simulink model "SOC_uncommented"  (R2023b) (continued)
== system SOC_uncommented/SOC == (continued)
  Multi-Temperature SOC EKF69 : Subsystem  TreatAsAtomicUnit=on  SystemSampleTime=1
  Multi-Temperature SOC EKF7 : Subsystem  TreatAsAtomicUnit=on  SystemSampleTime=1
  Multi-Temperature SOC EKF70 : Subsystem  TreatAsAtomicUnit=on  SystemSampleTime=1
  Multi-Temperature SOC EKF71 : Subsystem  TreatAsAtomicUnit=on  SystemSampleTime=1
  Multi-Temperature SOC EKF72 : Subsystem  TreatAsAtomicUnit=on  SystemSampleTime=1
  Multi-Temperature SOC EKF73 : Subsystem  TreatAsAtomicUnit=on  SystemSampleTime=1
  Multi-Temperature SOC EKF74 : Subsystem  TreatAsAtomicUnit=on  SystemSampleTime=1
  Multi-Temperature SOC EKF75 : Subsystem  TreatAsAtomicUnit=on  SystemSampleTime=1
  Multi-Temperature SOC EKF76 : Subsystem  TreatAsAtomicUnit=on  SystemSampleTime=1
  Multi-Temperature SOC EKF77 : Subsystem  TreatAsAtomicUnit=on  SystemSampleTime=1
  Multi-Temperature SOC EKF78 : Subsystem  TreatAsAtomicUnit=on  SystemSampleTime=1
  Multi-Temperature SOC EKF79 : Subsystem  TreatAsAtomicUnit=on  SystemSampleTime=1
  Multi-Temperature SOC EKF8 : Subsystem  TreatAsAtomicUnit=on  SystemSampleTime=1
  Multi-Temperature SOC EKF80 : Subsystem  TreatAsAtomicUnit=on  SystemSampleTime=1
  Multi-Temperature SOC EKF81 : Subsystem  TreatAsAtomicUnit=on  SystemSampleTime=1
  Multi-Temperature SOC EKF82 : Subsystem  TreatAsAtomicUnit=on  SystemSampleTime=1
  Multi-Temperature SOC EKF83 : Subsystem  TreatAsAtomicUnit=on  SystemSampleTime=1
  Multi-Temperature SOC EKF84 : Subsystem  TreatAsAtomicUnit=on  SystemSampleTime=1
  Multi-Temperature SOC EKF85 : Subsystem  TreatAsAtomicUnit=on  SystemSampleTime=1
  Multi-Temperature SOC EKF86 : Subsystem  TreatAsAtomicUnit=on  SystemSampleTime=1
  Multi-Temperature SOC EKF87 : Subsystem  TreatAsAtomicUnit=on  SystemSampleTime=1
  Multi-Temperature SOC EKF88 : Subsystem  TreatAsAtomicUnit=on  SystemSampleTime=1
  Multi-Temperature SOC EKF89 : Subsystem  TreatAsAtomicUnit=on  SystemSampleTime=1
  Multi-Temperature SOC EKF9 : Subsystem  TreatAsAtomicUnit=on  SystemSampleTime=1
  Multi-Temperature SOC EKF90 : Subsystem  TreatAsAtomicUnit=on  SystemSampleTime=1
  Multi-Temperature SOC EKF91 : Subsystem  TreatAsAtomicUnit=on  SystemSampleTime=1
  Multi-Temperature SOC EKF92 : Subsystem  TreatAsAtomicUnit=on  SystemSampleTime=1
  Multi-Temperature SOC EKF93 : Subsystem  TreatAsAtomicUnit=on  SystemSampleTime=1
  Multi-Temperature SOC EKF94 : Subsystem  TreatAsAtomicUnit=on  SystemSampleTime=1
  Multi-Temperature SOC EKF95 : Subsystem  TreatAsAtomicUnit=on  SystemSampleTime=1
  Multi-Temperature SOC EKF96 : Subsystem  TreatAsAtomicUnit=on  SystemSampleTime=1
  Multi-Temperature SOC EKF97 : Subsystem  TreatAsAtomicUnit=on  SystemSampleTime=1
  Multi-Temperature SOC EKF98 : Subsystem  TreatAsAtomicUnit=on  SystemSampleTime=1
  Multi-Temperature SOC EKF99 : Subsystem  TreatAsAtomicUnit=on  SystemSampleTime=1
  tag scope: HVBatSOC=global, HVBatSOCMax=global, HVBatSOCMin=global, HVBat_SOCcells=global
  wires:
  Bus Selector2/63 -> Multi-Temperature SOC EKF63/3(Temperature)  "<Cell Temperature 63>"
  Bus Selector2/81 -> Multi-Temperature SOC EKF81/3(Temperature)  "<Cell Temperature 81>"
  Bus Selector2/101 -> Multi-Temperature SOC EKF101/3(Temperature)  "<Cell Temperature 101>"
  [HVBatCell_Voltage042] -> Multi-Temperature SOC EKF42/2(Voltage)
  [HVBatCell_Voltage095] -> Multi-Temperature SOC EKF95/2(Voltage)
  Bus Selector2/70 -> Multi-Temperature SOC EKF70/3(Temperature)  "<Cell Temperature 70>"
  Multi-Temperature SOC EKF76/1(HVBat_SOCcell076) -> Bus Creator2/76  "HVBat_SOCcell076"
  [HVBatCell_Voltage055] -> Multi-Temperature SOC EKF55/2(Voltage)
  Bus Selector2/82 -> Multi-Temperature SOC EKF82/3(Temperature)  "<Cell Temperature 82>"
  Bus Selector2/83 -> Multi-Temperature SOC EKF83/3(Temperature)  "<Cell Temperature 83>"
  Bus Selector2/85 -> Multi-Temperature SOC EKF85/3(Temperature)  "<Cell Temperature 85>"
  Bus Selector2/10 -> Multi-Temperature SOC EKF10/3(Temperature)  "<Cell Temperature 10>"
  [HVBatCell_Voltage077] -> Multi-Temperature SOC EKF77/2(Voltage)
  [HVBatCell_Voltage088] -> Multi-Temperature SOC EKF88/2(Voltage)
  Multi-Temperature SOC EKF28/1(HVBat_SOCcell028) -> Bus Creator2/28  "HVBat_SOCcell028"
  [HVBatCell_Voltage020] -> Multi-Temperature SOC EKF20/2(Voltage)
  Bus Selector2/100 -> Multi-Temperature SOC EKF100/3(Temperature)  "<Cell Temperature 100>"
  Multi-Temperature SOC EKF12/1(HVBat_SOCcell012) -> Bus Creator2/12  "HVBat_SOCcell012"
  [HVBatCell_Voltage089] -> Multi-Temperature SOC EKF89/2(Voltage)
  Bus Selector2/18 -> Multi-Temperature SOC EKF18/3(Temperature)  "<Cell Temperature 18>"
  [HVBatCell_Voltage029] -> Multi-Temperature SOC EKF29/2(Voltage)
  [HVBatCell_Voltage015] -> Multi-Temperature SOC EKF15/2(Voltage)
  [HVBatCell_Voltage075] -> Multi-Temperature SOC EKF75/2(Voltage)
  Bus Selector2/20 -> Multi-Temperature SOC EKF20/3(Temperature)  "<Cell Temperature 20>"
  [HVBatCell_Voltage102] -> Multi-Temperature SOC EKF102/2(Voltage)
  [HVBatCell_Voltage091] -> Multi-Temperature SOC EKF91/2(Voltage)
  Multi-Temperature SOC EKF41/1(HVBat_SOCcell041) -> Bus Creator2/41  "HVBat_SOCcell041"
  Bus Selector2/71 -> Multi-Temperature SOC EKF71/3(Temperature)  "<Cell Temperature 71>"
  [HVBatCell_Voltage092] -> Multi-Temperature SOC EKF92/2(Voltage)
  Multi-Temperature SOC EKF50/1(HVBat_SOCcell050) -> Bus Creator2/50  "HVBat_SOCcell050"
  Multi-Temperature SOC EKF15/1(HVBat_SOCcell015) -> Bus Creator2/15  "HVBat_SOCcell015"
  [HVBatCell_Voltage048] -> Multi-Temperature SOC EKF48/2(Voltage)
  [HVBatCell_Voltage041] -> Multi-Temperature SOC EKF41/2(Voltage)
  Bus Selector2/49 -> Multi-Temperature SOC EKF49/3(Temperature)  "<Cell Temperature 49>"
  Bus Selector2/50 -> Multi-Temperature SOC EKF50/3(Temperature)  "<Cell Temperature 50>"
  [HVBatCell_Voltage093] -> Multi-Temperature SOC EKF93/2(Voltage)
  Bus Selector2/37 -> Multi-Temperature SOC EKF37/3(Temperature)  "<Cell Temperature 37>"
  Bus Selector2/2 -> Multi-Temperature SOC EKF2/3(Temperature)  "<Cell Temperature 2>"
  Gain1/1 -> Multi-Temperature SOC EKF2/1(Current), Multi-Temperature SOC EKF34/1(Current), Multi-Temperature SOC EKF35/1(Current), Multi-Temperature SOC EKF39/1(Current), Multi-Temperature SOC EKF105/1(Current), Multi-Temperature SOC EKF104/1(Current), Multi-Temperature SOC EKF103/1(Current), Multi-Temperature SOC EKF102/1(Current), Multi-Temperature SOC EKF101/1(Current), Multi-Temperature SOC EKF100/1(Current), Multi-Temperature SOC EKF99/1(Current), Multi-Temperature SOC EKF98/1(Current), Multi-Temperature SOC EKF97/1(Current), Multi-Temperature SOC EKF96/1(Current), Multi-Temperature SOC EKF95/1(Current), Multi-Temperature SOC EKF94/1(Current), Multi-Temperature SOC EKF93/1(Current), Multi-Temperature SOC EKF92/1(Current), Multi-Temperature SOC EKF91/1(Current), Multi-Temperature SOC EKF90/1(Current), Multi-Temperature SOC EKF89/1(Current), Multi-Temperature SOC EKF88/1(Current), Multi-Temperature SOC EKF87/1(Current), Multi-Temperature SOC EKF86/1(Current), Multi-Temperature SOC EKF85/1(Current), Multi-Temperature SOC EKF84/1(Current), Multi-Temperature SOC EKF83/1(Current), Multi-Temperature SOC EKF82/1(Current), Multi-Temperature SOC EKF81/1(Current), Multi-Temperature SOC EKF80/1(Current), Multi-Temperature SOC EKF79/1(Current), Multi-Temperature SOC EKF78/1(Current), Multi-Temperature SOC EKF77/1(Current), Multi-Temperature SOC EKF76/1(Current), Multi-Temperature SOC EKF75/1(Current), Multi-Temperature SOC EKF74/1(Current), Multi-Temperature SOC EKF73/1(Current), Multi-Temperature SOC EKF72/1(Current), Multi-Temperature SOC EKF71/1(Current), Multi-Temperature SOC EKF70/1(Current), Multi-Temperature SOC EKF69/1(Current), Multi-Temperature SOC EKF68/1(Current), Multi-Temperature SOC EKF67/1(Current), Multi-Temperature SOC EKF66/1(Current), Multi-Temperature SOC EKF65/1(Current), Multi-Temperature SOC EKF64/1(Current), Multi-Temperature SOC EKF63/1(Current), Multi-Temperature SOC EKF62/1(Current), Multi-Temperature SOC EKF61/1(Current), Multi-Temperature SOC EKF60/1(Current), Multi-Temperature SOC EKF59/1(Current), Multi-Temperature SOC EKF58/1(Current), Multi-Temperature SOC EKF57/1(Current), Multi-Temperature SOC EKF56/1(Current), Multi-Temperature SOC EKF55/1(Current), Multi-Temperature SOC EKF54/1(Current), Multi-Temperature SOC EKF53/1(Current), Multi-Temperature SOC EKF52/1(Current), Multi-Temperature SOC EKF51/1(Current), Multi-Temperature SOC EKF50/1(Current), Multi-Temperature SOC EKF49/1(Current), Multi-Temperature SOC EKF48/1(Current), Multi-Temperature SOC EKF47/1(Current), Multi-Temperature SOC EKF46/1(Current), Multi-Temperature SOC EKF45/1(Current), Multi-Temperature SOC EKF44/1(Current), Multi-Temperature SOC EKF43/1(Current), Multi-Temperature SOC EKF42/1(Current), Multi-Temperature SOC EKF41/1(Current), Multi-Temperature SOC EKF40/1(Current), Multi-Temperature SOC EKF38/1(Current), Multi-Temperature SOC EKF37/1(Current), Multi-Temperature SOC EKF36/1(Current), Multi-Temperature SOC EKF33/1(Current), Multi-Temperature SOC EKF32/1(Current), Multi-Temperature SOC EKF31/1(Current), Multi-Temperature SOC EKF30/1(Current), Multi-Temperature SOC EKF29/1(Current), Multi-Temperature SOC EKF28/1(Current), Multi-Temperature SOC EKF27/1(Current), Multi-Temperature SOC EKF26/1(Current), Multi-Temperature SOC EKF25/1(Current), Multi-Temperature SOC EKF24/1(Current), Multi-Temperature SOC EKF23/1(Current), Multi-Temperature SOC EKF22/1(Current), Multi-Temperature SOC EKF21/1(Current), Multi-Temperature SOC EKF20/1(Current), Multi-Temperature SOC EKF19/1(Current), Multi-Temperature SOC EKF18/1(Current), Multi-Temperature SOC EKF17/1(Current), Multi-Temperature SOC EKF16/1(Current), Multi-Temperature SOC EKF15/1(Current), Multi-Temperature SOC EKF14/1(Current), Multi-Temperature SOC EKF13/1(Current), Multi-Temperature SOC EKF12/1(Current), Multi-Temperature SOC EKF11/1(Current), Multi-Temperature SOC EKF10/1(Current), Multi-Temperature SOC EKF9/1(Current), Multi-Temperature SOC EKF8/1(Current), Multi-Temperature SOC EKF7/1(Current), Multi-Temperature SOC EKF6/1(Current), Multi-Temperature SOC EKF5/1(Current), Multi-Temperature SOC EKF4/1(Current), Multi-Temperature SOC EKF3/1(Current), Multi-Temperature SOC EKF1/1(Current)  "CellCurrent"
  Multi-Temperature SOC EKF102/1(HVBat_SOCcell102) -> Bus Creator2/102  "HVBat_SOCcell102"
  Multi-Temperature SOC EKF54/1(HVBat_SOCcell054) -> Bus Creator2/54  "HVBat_SOCcell054"
  Bus Selector2/75 -> Multi-Temperature SOC EKF75/3(Temperature)  "<Cell Temperature 75>"
  [HVBatCell_Voltage007] -> Multi-Temperature SOC EKF7/2(Voltage)
  Multi-Temperature SOC EKF11/1(HVBat_SOCcell011) -> Bus Creator2/11  "HVBat_SOCcell011"
  [HVBatCell_Voltage087] -> Multi-Temperature SOC EKF87/2(Voltage)
  Multi-Temperature SOC EKF4/1(HVBat_SOCcell004) -> Bus Creator2/4  "HVBat_SOCcell004"
  [HVBatCell_Voltage037] -> Multi-Temperature SOC EKF37/2(Voltage)
  Bus Selector2/57 -> Multi-Temperature SOC EKF57/3(Temperature)  "<Cell Temperature 57>"
  [HVBatCell_Voltage083] -> Multi-Temperature SOC EKF83/2(Voltage)
  Bus Selector2/43 -> Multi-Temperature SOC EKF43/3(Temperature)  "<Cell Temperature 43>"
  [HVBatCell_Voltage035] -> Multi-Temperature SOC EKF35/2(Voltage)
  [HVBatCell_Voltage053] -> Multi-Temperature SOC EKF53/2(Voltage)
  Multi-Temperature SOC EKF35/1(HVBat_SOCcell035) -> Bus Creator2/35  "HVBat_SOCcell035"
  Multi-Temperature SOC EKF65/1(HVBat_SOCcell065) -> Bus Creator2/65  "HVBat_SOCcell065"
  Multi-Temperature SOC EKF73/1(HVBat_SOCcell073) -> Bus Creator2/73  "HVBat_SOCcell073"
  [HVBatCell_Voltage001] -> Multi-Temperature SOC EKF1/2(Voltage)
  [HVBatCell_Voltage084] -> Multi-Temperature SOC EKF84/2(Voltage)
  [HVBatCell_Voltage098] -> Multi-Temperature SOC EKF98/2(Voltage)
  Bus Selector2/62 -> Multi-Temperature SOC EKF62/3(Temperature)  "<Cell Temperature 62>"
  [HVBatCell_Voltage034] -> Multi-Temperature SOC EKF34/2(Voltage)
  Bus Selector2/5 -> Multi-Temperature SOC EKF5/3(Temperature)  "<Cell Temperature 5>"
  Multi-Temperature SOC EKF67/1(HVBat_SOCcell067) -> Bus Creator2/67  "HVBat_SOCcell067"
  [HVBatCell_Temperatures] -> Bus Selector2/1
  [HVBatCell_Voltage104] -> Multi-Temperature SOC EKF104/2(Voltage)
  Bus Selector2/3 -> Multi-Temperature SOC EKF3/3(Temperature)  "<Cell Temperature 3>"
  Bus Selector2/29 -> Multi-Temperature SOC EKF29/3(Temperature)  "<Cell Temperature 29>"
  Multi-Temperature SOC EKF78/1(HVBat_SOCcell078) -> Bus Creator2/78  "HVBat_SOCcell078"
  Multi-Temperature SOC EKF104/1(HVBat_SOCcell104) -> Bus Creator2/104  "HVBat_SOCcell104"
  Multi-Temperature SOC EKF46/1(HVBat_SOCcell046) -> Bus Creator2/46  "HVBat_SOCcell046"
  Bus Selector2/95 -> Multi-Temperature SOC EKF95/3(Temperature)  "<Cell Temperature 95>"
  [HVBatCell_Voltage023] -> Multi-Temperature SOC EKF23/2(Voltage)
  [HVBatCell_Voltage019] -> Multi-Temperature SOC EKF19/2(Voltage)
  Bus Selector2/51 -> Multi-Temperature SOC EKF51/3(Temperature)  "<Cell Temperature 51>"
  [HVBatCell_Voltage078] -> Multi-Temperature SOC EKF78/2(Voltage)
  Bus Selector2/65 -> Multi-Temperature SOC EKF65/3(Temperature)  "<Cell Temperature 65>"
  Multi-Temperature SOC EKF6/1(HVBat_SOCcell006) -> Bus Creator2/6  "HVBat_SOCcell006"
  Multi-Temperature SOC EKF103/1(HVBat_SOCcell103) -> Bus Creator2/103  "HVBat_SOCcell103"
  Bus Selector2/64 -> Multi-Temperature SOC EKF64/3(Temperature)  "<Cell Temperature 64>"
  [HVBatCell_Voltage052] -> Multi-Temperature SOC EKF52/2(Voltage)
  Multi-Temperature SOC EKF38/1(HVBat_SOCcell038) -> Bus Creator2/38  "HVBat_SOCcell038"
  Multi-Temperature SOC EKF30/1(HVBat_SOCcell030) -> Bus Creator2/30  "HVBat_SOCcell030"
  [HVBatCell_Voltage054] -> Multi-Temperature SOC EKF54/2(Voltage)
  Bus Selector2/45 -> Multi-Temperature SOC EKF45/3(Temperature)  "<Cell Temperature 45>"
  [HVBatCell_Voltage081] -> Multi-Temperature SOC EKF81/2(Voltage)
  Bus Selector2/26 -> Multi-Temperature SOC EKF26/3(Temperature)  "<Cell Temperature 26>"
  Bus Selector2/4 -> Multi-Temperature SOC EKF4/3(Temperature)  "<Cell Temperature 4>"
  [HVBatCell_Voltage049] -> Multi-Temperature SOC EKF49/2(Voltage)
  Multi-Temperature SOC EKF95/1(HVBat_SOCcell095) -> Bus Creator2/95  "HVBat_SOCcell095"
  Multi-Temperature SOC EKF100/1(HVBat_SOCcell100) -> Bus Creator2/100  "HVBat_SOCcell100"
  [HVBatCell_Voltage067] -> Multi-Temperature SOC EKF67/2(Voltage)
  [HVBatCell_Voltage069] -> Multi-Temperature SOC EKF69/2(Voltage)
  Bus Selector2/12 -> Multi-Temperature SOC EKF12/3(Temperature)  "<Cell Temperature 12>"
  Bus Selector2/93 -> Multi-Temperature SOC EKF93/3(Temperature)  "<Cell Temperature 93>"
  Multi-Temperature SOC EKF7/1(HVBat_SOCcell007) -> Bus Creator2/7  "HVBat_SOCcell007"
  Bus Selector2/91 -> Multi-Temperature SOC EKF91/3(Temperature)  "<Cell Temperature 91>"
  [HVBatCell_Voltage099] -> Multi-Temperature SOC EKF99/2(Voltage)
  [HVBatCell_Voltage032] -> Multi-Temperature SOC EKF32/2(Voltage)
  [HVBatCell_Voltage051] -> Multi-Temperature SOC EKF51/2(Voltage)
  Multi-Temperature SOC EKF16/1(HVBat_SOCcell016) -> Bus Creator2/16  "HVBat_SOCcell016"
  [HVBatCell_Voltage080] -> Multi-Temperature SOC EKF80/2(Voltage)
  [HVBatCell_Voltage021] -> Multi-Temperature SOC EKF21/2(Voltage)
  Multi-Temperature SOC EKF2/1(HVBat_SOCcell002) -> Bus Creator2/2  "HVBat_SOCcell002"
  Multi-Temperature SOC EKF27/1(HVBat_SOCcell027) -> Bus Creator2/27  "HVBat_SOCcell027"
  Bus Selector2/72 -> Multi-Temperature SOC EKF72/3(Temperature)  "<Cell Temperature 72>"
  Bus Selector2/80 -> Multi-Temperature SOC EKF80/3(Temperature)  "<Cell Temperature 80>"
  [HVBatCell_Voltage031] -> Multi-Temperature SOC EKF31/2(Voltage)
  [HVBatCell_Voltage045] -> Multi-Temperature SOC EKF45/2(Voltage)
  MATLAB Function1/2(HVBat_Energy) -> Data Store Write3/1
  Bus Selector2/56 -> Multi-Temperature SOC EKF56/3(Temperature)  "<Cell Temperature 56>"
  [HVBatCell_Voltage047] -> Multi-Temperature SOC EKF47/2(Voltage)
  Bus Selector2/97 -> Multi-Temperature SOC EKF97/3(Temperature)  "<Cell Temperature 97>"
  Bus Selector2/44 -> Multi-Temperature SOC EKF44/3(Temperature)  "<Cell Temperature 44>"
  Multi-Temperature SOC EKF47/1(HVBat_SOCcell047) -> Bus Creator2/47  "HVBat_SOCcell047"
  Bus Selector2/74 -> Multi-Temperature SOC EKF74/3(Temperature)  "<Cell Temperature 74>"
  Bus Selector2/90 -> Multi-Temperature SOC EKF90/3(Temperature)  "<Cell Temperature 90>"
  [HVBatCell_Voltage003] -> Multi-Temperature SOC EKF3/2(Voltage)
  Multi-Temperature SOC EKF86/1(HVBat_SOCcell086) -> Bus Creator2/86  "HVBat_SOCcell086"
  Bus Selector2/98 -> Multi-Temperature SOC EKF98/3(Temperature)  "<Cell Temperature 98>"
  Multi-Temperature SOC EKF20/1(HVBat_SOCcell020) -> Bus Creator2/20  "HVBat_SOCcell020"
  Multi-Temperature SOC EKF13/1(HVBat_SOCcell013) -> Bus Creator2/13  "HVBat_SOCcell013"
  [HVBatCell_Voltage100] -> Multi-Temperature SOC EKF100/2(Voltage)
  [HVBatCell_Voltage074] -> Multi-Temperature SOC EKF74/2(Voltage)
  Multi-Temperature SOC EKF19/1(HVBat_SOCcell019) -> Bus Creator2/19  "HVBat_SOCcell019"
  [HVBatCell_Voltage009] -> Multi-Temperature SOC EKF9/2(Voltage)
  Multi-Temperature SOC EKF39/1(HVBat_SOCcell039) -> Bus Creator2/39  "HVBat_SOCcell039"
  [HVBatCell_Voltage070] -> Multi-Temperature SOC EKF70/2(Voltage)
  Max1/1 -> [HVBatSOCMin], MATLAB Function/2(HVBatSOCMin)  "HVBatSOCMin"
  [HVBatCell_Voltage094] -> Multi-Temperature SOC EKF94/2(Voltage)
  Multi-Temperature SOC EKF5/1(HVBat_SOCcell005) -> Bus Creator2/5  "HVBat_SOCcell005"
  [HVBatCell_Voltage050] -> Multi-Temperature SOC EKF50/2(Voltage)
  Bus Selector2/52 -> Multi-Temperature SOC EKF52/3(Temperature)  "<Cell Temperature 52>"
  [HVBatCell_Voltage059] -> Multi-Temperature SOC EKF59/2(Voltage)
  [HVBatCell_Voltage010] -> Multi-Temperature SOC EKF10/2(Voltage)
  Multi-Temperature SOC EKF25/1(HVBat_SOCcell025) -> Bus Creator2/25  "HVBat_SOCcell025"
  [HVBatCell_Voltage013] -> Multi-Temperature SOC EKF13/2(Voltage)
  Bus Selector2/99 -> Multi-Temperature SOC EKF99/3(Temperature)  "<Cell Temperature 99>"
  Multi-Temperature SOC EKF105/1(HVBat_SOCcell105) -> Bus Creator2/105  "HVBat_SOCcell105"
  [HVBatCell_Voltage011] -> Multi-Temperature SOC EKF11/2(Voltage)
  Multi-Temperature SOC EKF18/1(HVBat_SOCcell018) -> Bus Creator2/18  "HVBat_SOCcell018"
  Multi-Temperature SOC EKF43/1(HVBat_SOCcell043) -> Bus Creator2/43  "HVBat_SOCcell043"
  Multi-Temperature SOC EKF68/1(HVBat_SOCcell068) -> Bus Creator2/68  "HVBat_SOCcell068"
  Bus Selector2/67 -> Multi-Temperature SOC EKF67/3(Temperature)  "<Cell Temperature 67>"
  Multi-Temperature SOC EKF98/1(HVBat_SOCcell098) -> Bus Creator2/98  "HVBat_SOCcell098"
  [HVBatCell_Voltage097] -> Multi-Temperature SOC EKF97/2(Voltage)
  Multi-Temperature SOC EKF94/1(HVBat_SOCcell094) -> Bus Creator2/94  "HVBat_SOCcell094"
  [HVBatCell_Voltage026] -> Multi-Temperature SOC EKF26/2(Voltage)
  Multi-Temperature SOC EKF42/1(HVBat_SOCcell042) -> Bus Creator2/42  "HVBat_SOCcell042"
  Multi-Temperature SOC EKF90/1(HVBat_SOCcell090) -> Bus Creator2/90  "HVBat_SOCcell090"
  Bus Selector2/68 -> Multi-Temperature SOC EKF68/3(Temperature)  "<Cell Temperature 68>"
  Multi-Temperature SOC EKF93/1(HVBat_SOCcell093) -> Bus Creator2/93  "HVBat_SOCcell093"
  Multi-Temperature SOC EKF91/1(HVBat_SOCcell091) -> Bus Creator2/91  "HVBat_SOCcell091"
  Multi-Temperature SOC EKF87/1(HVBat_SOCcell087) -> Bus Creator2/87  "HVBat_SOCcell087"
  [HVBatCell_Voltage016] -> Multi-Temperature SOC EKF16/2(Voltage)
  [HVBatCell_Voltage096] -> Multi-Temperature SOC EKF96/2(Voltage)
  Multi-Temperature SOC EKF34/1(HVBat_SOCcell034) -> Bus Creator2/34  "HVBat_SOCcell034"
  Multi-Temperature SOC EKF62/1(HVBat_SOCcell062) -> Bus Creator2/62  "HVBat_SOCcell062"
  Multi-Temperature SOC EKF45/1(HVBat_SOCcell045) -> Bus Creator2/45  "HVBat_SOCcell045"
  Multi-Temperature SOC EKF60/1(HVBat_SOCcell060) -> Bus Creator2/60  "HVBat_SOCcell060"
  [HVBatCell_Voltage027] -> Multi-Temperature SOC EKF27/2(Voltage)
  Multi-Temperature SOC EKF26/1(HVBat_SOCcell026) -> Bus Creator2/26  "HVBat_SOCcell026"
  Multi-Temperature SOC EKF8/1(HVBat_SOCcell008) -> Bus Creator2/8  "HVBat_SOCcell008"
  [HVBatCell_Voltage082] -> Multi-Temperature SOC EKF82/2(Voltage)
  [HVBatCell_Voltage073] -> Multi-Temperature SOC EKF73/2(Voltage)
  Multi-Temperature SOC EKF17/1(HVBat_SOCcell017) -> Bus Creator2/17  "HVBat_SOCcell017"
  Multi-Temperature SOC EKF88/1(HVBat_SOCcell088) -> Bus Creator2/88  "HVBat_SOCcell088"
  Bus Selector2/104 -> Multi-Temperature SOC EKF104/3(Temperature)  "<Cell Temperature 87>"
  Multi-Temperature SOC EKF69/1(HVBat_SOCcell069) -> Bus Creator2/69  "HVBat_SOCcell069"
  Bus Selector2/69 -> Multi-Temperature SOC EKF69/3(Temperature)  "<Cell Temperature 69>"
  Bus Selector2/34 -> Multi-Temperature SOC EKF34/3(Temperature)  "<Cell Temperature 34>"
  [HVBatCell_Voltage105] -> Multi-Temperature SOC EKF105/2(Voltage)
  Bus Selector2/61 -> Multi-Temperature SOC EKF61/3(Temperature)  "<Cell Temperature 61>"
  Multi-Temperature SOC EKF23/1(HVBat_SOCcell023) -> Bus Creator2/23  "HVBat_SOCcell023"
  Bus Selector2/84 -> Multi-Temperature SOC EKF84/3(Temperature)  "<Cell Temperature 84>"
  [HVBatCell_Voltage064] -> Multi-Temperature SOC EKF64/2(Voltage)
  Bus Selector2/60 -> Multi-Temperature SOC EKF60/3(Temperature)  "<Cell Temperature 60>"
  Bus Selector2/59 -> Multi-Temperature SOC EKF59/3(Temperature)  "<Cell Temperature 59>"
  Multi-Temperature SOC EKF79/1(HVBat_SOCcell079) -> Bus Creator2/79  "HVBat_SOCcell079"
  Multi-Temperature SOC EKF75/1(HVBat_SOCcell075) -> Bus Creator2/75  "HVBat_SOCcell075"
  Bus Selector2/103 -> Multi-Temperature SOC EKF103/3(Temperature)  "<Cell Temperature 103>"
  Bus Selector2/24 -> Multi-Temperature SOC EKF24/3(Temperature)  "<Cell Temperature 24>"
  Multi-Temperature SOC EKF14/1(HVBat_SOCcell014) -> Bus Creator2/14  "HVBat_SOCcell014"
  [HVBatCell_Voltage079] -> Multi-Temperature SOC EKF79/2(Voltage)
  Max4/1 -> [HVBatSOCMax], MATLAB Function/1(HVBatSOCMax)  "HVBatSOCMax"
  [HVBatCell_Voltage057] -> Multi-Temperature SOC EKF57/2(Voltage)
  Bus Selector2/27 -> Multi-Temperature SOC EKF27/3(Temperature)  "<Cell Temperature 27>"
  Bus Selector2/14 -> Multi-Temperature SOC EKF14/3(Temperature)  "<Cell Temperature 14>"
  Multi-Temperature SOC EKF81/1(HVBat_SOCcell081) -> Bus Creator2/81  "HVBat_SOCcell081"
  Multi-Temperature SOC EKF29/1(HVBat_SOCcell029) -> Bus Creator2/29  "HVBat_SOCcell029"
  Bus Selector2/39 -> Multi-Temperature SOC EKF39/3(Temperature)  "<Cell Temperature 39>"
  Multi-Temperature SOC EKF33/1(HVBat_SOCcell033) -> Bus Creator2/33  "HVBat_SOCcell033"
  Multi-Temperature SOC EKF92/1(HVBat_SOCcell092) -> Bus Creator2/92  "HVBat_SOCcell092"
  Bus Selector2/30 -> Multi-Temperature SOC EKF30/3(Temperature)  "<Cell Temperature 30>"
  [HVBatCell_Voltage038] -> Multi-Temperature SOC EKF38/2(Voltage)
  Bus Selector2/89 -> Multi-Temperature SOC EKF89/3(Temperature)  "<Cell Temperature 89>"
  Bus Selector2/36 -> Multi-Temperature SOC EKF36/3(Temperature)  "<Cell Temperature 36>"
  Multi-Temperature SOC EKF22/1(HVBat_SOCcell022) -> Bus Creator2/22  "HVBat_SOCcell022"
  Bus Selector2/55 -> Multi-Temperature SOC EKF55/3(Temperature)  "<Cell Temperature 55>"
  Multi-Temperature SOC EKF84/1(HVBat_SOCcell084) -> Bus Creator2/84  "HVBat_SOCcell084"
  Multi-Temperature SOC EKF9/1(HVBat_SOCcell009) -> Bus Creator2/9  "HVBat_SOCcell009"
  Multi-Temperature SOC EKF85/1(HVBat_SOCcell085) -> Bus Creator2/85  "HVBat_SOCcell085"
  Bus Creator2/1 -> Max1/1, Max4/1, [HVBat_SOCcells]
  Bus Selector2/73 -> Multi-Temperature SOC EKF73/3(Temperature)  "<Cell Temperature 73>"
  Multi-Temperature SOC EKF3/1(HVBat_SOCcell003) -> Bus Creator2/3  "HVBat_SOCcell003"
  Multi-Temperature SOC EKF77/1(HVBat_SOCcell077) -> Bus Creator2/77  "HVBat_SOCcell077"
  Multi-Temperature SOC EKF48/1(HVBat_SOCcell048) -> Bus Creator2/48  "HVBat_SOCcell048"
  Multi-Temperature SOC EKF37/1(HVBat_SOCcell037) -> Bus Creator2/37  "HVBat_SOCcell037"
  [HVBatCell_Voltage043] -> Multi-Temperature SOC EKF43/2(Voltage)
  Bus Selector2/46 -> Multi-Temperature SOC EKF46/3(Temperature)  "<Cell Temperature 46>"
  [HVBatPack_Current_Prim] -> Gain1/1  "HVBatPack_Current_Prim"
  [HVBatCell_Voltage086] -> Multi-Temperature SOC EKF86/2(Voltage)
  Multi-Temperature SOC EKF56/1(HVBat_SOCcell056) -> Bus Creator2/56  "HVBat_SOCcell056"
  Multi-Temperature SOC EKF71/1(HVBat_SOCcell071) -> Bus Creator2/71  "HVBat_SOCcell071"
  [HVBatCell_Voltage036] -> Multi-Temperature SOC EKF36/2(Voltage)
  [HVBatCell_Voltage025] -> Multi-Temperature SOC EKF25/2(Voltage)
  [HVBatCell_Voltage044] -> Multi-Temperature SOC EKF44/2(Voltage)
  Multi-Temperature SOC EKF59/1(HVBat_SOCcell059) -> Bus Creator2/59  "HVBat_SOCcell059"
  Multi-Temperature SOC EKF53/1(HVBat_SOCcell053) -> Bus Creator2/53  "HVBat_SOCcell053"
  Bus Selector2/88 -> Multi-Temperature SOC EKF88/3(Temperature)  "<Cell Temperature 88>"
  Multi-Temperature SOC EKF97/1(HVBat_SOCcell097) -> Bus Creator2/97  "HVBat_SOCcell097"
  Multi-Temperature SOC EKF58/1(HVBat_SOCcell058) -> Bus Creator2/58  "HVBat_SOCcell058"
  Multi-Temperature SOC EKF44/1(HVBat_SOCcell044) -> Bus Creator2/44  "HVBat_SOCcell044"
  Bus Selector2/35 -> Multi-Temperature SOC EKF35/3(Temperature)  "<Cell Temperature 35>"
  [HVBatCell_Voltage022] -> Multi-Temperature SOC EKF22/2(Voltage)
  [HVBatCell_Voltage030] -> Multi-Temperature SOC EKF30/2(Voltage)
  Bus Selector2/33 -> Multi-Temperature SOC EKF33/3(Temperature)  "<Cell Temperature 33>"
  Multi-Temperature SOC EKF63/1(HVBat_SOCcell063) -> Bus Creator2/63  "HVBat_SOCcell063"
  Multi-Temperature SOC EKF66/1(HVBat_SOCcell066) -> Bus Creator2/66  "HVBat_SOCcell066"
  Bus Selector2/42 -> Multi-Temperature SOC EKF42/3(Temperature)  "<Cell Temperature 42>"
  Multi-Temperature SOC EKF32/1(HVBat_SOCcell032) -> Bus Creator2/32  "HVBat_SOCcell032"
  Bus Selector2/79 -> Multi-Temperature SOC EKF79/3(Temperature)  "<Cell Temperature 79>"
  Multi-Temperature SOC EKF70/1(HVBat_SOCcell070) -> Bus Creator2/70  "HVBat_SOCcell070"
  Multi-Temperature SOC EKF49/1(HVBat_SOCcell049) -> Bus Creator2/49  "HVBat_SOCcell049"
  [HVBatCell_Voltage040] -> Multi-Temperature SOC EKF40/2(Voltage)
  [HVBatCell_Voltage101] -> Multi-Temperature SOC EKF101/2(Voltage)
  [HVBatCell_Voltage018] -> Multi-Temperature SOC EKF18/2(Voltage)
  Multi-Temperature SOC EKF40/1(HVBat_SOCcell040) -> Bus Creator2/40  "HVBat_SOCcell040"
  Bus Selector2/19 -> Multi-Temperature SOC EKF19/3(Temperature)  "<Cell Temperature 19>"
  Multi-Temperature SOC EKF96/1(HVBat_SOCcell096) -> Bus Creator2/96  "HVBat_SOCcell096"
  Bus Selector2/54 -> Multi-Temperature SOC EKF54/3(Temperature)  "<Cell Temperature 54>"
  [HVBatCell_Voltage004] -> Multi-Temperature SOC EKF4/2(Voltage)
  [HVBatCell_Voltage076] -> Multi-Temperature SOC EKF76/2(Voltage)
  [HVBatCell_Voltage090] -> Multi-Temperature SOC EKF90/2(Voltage)
  [HVBatCell_Voltage033] -> Multi-Temperature SOC EKF33/2(Voltage)
  Multi-Temperature SOC EKF82/1(HVBat_SOCcell082) -> Bus Creator2/82  "HVBat_SOCcell082"
  Bus Selector2/47 -> Multi-Temperature SOC EKF47/3(Temperature)  "<Cell Temperature 47>"
  [HVBatCell_Voltage005] -> Multi-Temperature SOC EKF5/2(Voltage)
  Bus Selector2/25 -> Multi-Temperature SOC EKF25/3(Temperature)  "<Cell Temperature 25>"
  Multi-Temperature SOC EKF51/1(HVBat_SOCcell051) -> Bus Creator2/51  "HVBat_SOCcell051"
  [HVBatCell_Voltage066] -> Multi-Temperature SOC EKF66/2(Voltage)
  [HVBatCell_Voltage071] -> Multi-Temperature SOC EKF71/2(Voltage)
  Bus Selector2/21 -> Multi-Temperature SOC EKF21/3(Temperature)  "<Cell Temperature 21>"
  Multi-Temperature SOC EKF10/1(HVBat_SOCcell010) -> Bus Creator2/10  "HVBat_SOCcell010"
  Multi-Temperature SOC EKF31/1(HVBat_SOCcell031) -> Bus Creator2/31  "HVBat_SOCcell031"
  [HVBatCell_Voltage072] -> Multi-Temperature SOC EKF72/2(Voltage)
  Bus Selector2/13 -> Multi-Temperature SOC EKF13/3(Temperature)  "<Cell Temperature 13>"
  Multi-Temperature SOC EKF74/1(HVBat_SOCcell074) -> Bus Creator2/74  "HVBat_SOCcell074"
  Bus Selector2/1 -> Multi-Temperature SOC EKF1/3(Temperature)  "<Cell Temperature 1>"
  Multi-Temperature SOC EKF80/1(HVBat_SOCcell080) -> Bus Creator2/80  "HVBat_SOCcell080"
  [HVBatCell_Voltage061] -> Multi-Temperature SOC EKF61/2(Voltage)
  Multi-Temperature SOC EKF57/1(HVBat_SOCcell057) -> Bus Creator2/57  "HVBat_SOCcell057"
  Multi-Temperature SOC EKF83/1(HVBat_SOCcell083) -> Bus Creator2/83  "HVBat_SOCcell083"
  Multi-Temperature SOC EKF61/1(HVBat_SOCcell061) -> Bus Creator2/61  "HVBat_SOCcell061"
  [HVBatCell_Voltage103] -> Multi-Temperature SOC EKF103/2(Voltage)
  Bus Selector2/16 -> Multi-Temperature SOC EKF16/3(Temperature)  "<Cell Temperature 16>"
  [HVBatCell_Voltage024] -> Multi-Temperature SOC EKF24/2(Voltage)
  [HVBatPack_Voltage] -> MATLAB Function1/2(HVBatPack_Voltage)
  Multi-Temperature SOC EKF72/1(HVBat_SOCcell072) -> Bus Creator2/72  "HVBat_SOCcell072"
  Bus Selector2/76 -> Multi-Temperature SOC EKF76/3(Temperature)  "<Cell Temperature 76>"
  [HVBatCell_Voltage012] -> Multi-Temperature SOC EKF12/2(Voltage)
  Multi-Temperature SOC EKF101/1(HVBat_SOCcell101) -> Bus Creator2/101  "HVBat_SOCcell101"
  Bus Selector2/32 -> Multi-Temperature SOC EKF32/3(Temperature)  "<Cell Temperature 32>"
  Bus Selector2/7 -> Multi-Temperature SOC EKF7/3(Temperature)  "<Cell Temperature 7>"
  [HVBatCell_Voltage008] -> Multi-Temperature SOC EKF8/2(Voltage)
  [HVBatCell_Voltage014] -> Multi-Temperature SOC EKF14/2(Voltage)
  [HVBatCell_Voltage065] -> Multi-Temperature SOC EKF65/2(Voltage)
  Bus Selector2/15 -> Multi-Temperature SOC EKF15/3(Temperature)  "<Cell Temperature 15>"
  Bus Selector2/87 -> Multi-Temperature SOC EKF87/3(Temperature)  "<Cell Temperature 87>"
  Bus Selector2/9 -> Multi-Temperature SOC EKF9/3(Temperature)  "<Cell Temperature 9>"
  Bus Selector2/48 -> Multi-Temperature SOC EKF48/3(Temperature)  "<Cell Temperature 48>"
  [HVBatCell_Voltage068] -> Multi-Temperature SOC EKF68/2(Voltage)
  Bus Selector2/6 -> Multi-Temperature SOC EKF6/3(Temperature)  "<Cell Temperature 6>"
  [HVBatCell_Voltage028] -> Multi-Temperature SOC EKF28/2(Voltage)
  Bus Selector2/41 -> Multi-Temperature SOC EKF41/3(Temperature)  "<Cell Temperature 41>"
  Multi-Temperature SOC EKF52/1(HVBat_SOCcell052) -> Bus Creator2/52  "HVBat_SOCcell052"
  [HVBatCell_Voltage046] -> Multi-Temperature SOC EKF46/2(Voltage)
  [HVBatCell_Voltage063] -> Multi-Temperature SOC EKF63/2(Voltage)
  [HVBatCell_Voltage085] -> Multi-Temperature SOC EKF85/2(Voltage)
  MATLAB Function/1(HVBatSOC) -> MATLAB Function1/1(HVBatSOC), Data Store Write1/1, [HVBatSOC]  "HVBatSOC"
  Bus Selector2/66 -> Multi-Temperature SOC EKF66/3(Temperature)  "<Cell Temperature 66>"
  [HVBatCell_Voltage039] -> Multi-Temperature SOC EKF39/2(Voltage)
  Bus Selector2/31 -> Multi-Temperature SOC EKF31/3(Temperature)  "<Cell Temperature 31>"
  Multi-Temperature SOC EKF55/1(HVBat_SOCcell055) -> Bus Creator2/55  "HVBat_SOCcell055"
  Bus Selector2/78 -> Multi-Temperature SOC EKF78/3(Temperature)  "<Cell Temperature 78>"
  [HVBatCell_Voltage060] -> Multi-Temperature SOC EKF60/2(Voltage)
  MATLAB Function1/1(HVBatFull_Amp_Hr_Capacity) -> Data Store Write2/1
  Multi-Temperature SOC EKF1/1(HVBat_SOCcell001) -> Bus Creator2/1  "HVBat_SOCcell001"
  Bus Selector2/40 -> Multi-Temperature SOC EKF40/3(Temperature)  "<Cell Temperature 40>"
  Bus Selector2/58 -> Multi-Temperature SOC EKF58/3(Temperature)  "<Cell Temperature 58>"
  Bus Selector2/17 -> Multi-Temperature SOC EKF17/3(Temperature)  "<Cell Temperature 17>"
  Multi-Temperature SOC EKF89/1(HVBat_SOCcell089) -> Bus Creator2/89  "HVBat_SOCcell089"
  [HVBatCell_Voltage017] -> Multi-Temperature SOC EKF17/2(Voltage)
  Bus Selector2/8 -> Multi-Temperature SOC EKF8/3(Temperature)  "<Cell Temperature 8>"
  Bus Selector2/38 -> Multi-Temperature SOC EKF38/3(Temperature)  "<Cell Temperature 38>"
  Bus Selector2/23 -> Multi-Temperature SOC EKF23/3(Temperature)  "<Cell Temperature 23>"
  Bus Selector2/92 -> Multi-Temperature SOC EKF92/3(Temperature)  "<Cell Temperature 92>"
  [HVBatCell_Voltage062] -> Multi-Temperature SOC EKF62/2(Voltage)
  Multi-Temperature SOC EKF99/1(HVBat_SOCcell099) -> Bus Creator2/99  "HVBat_SOCcell099"
  Bus Selector2/53 -> Multi-Temperature SOC EKF53/3(Temperature)  "<Cell Temperature 53>"
  Multi-Temperature SOC EKF64/1(HVBat_SOCcell064) -> Bus Creator2/64  "HVBat_SOCcell064"
  Bus Selector2/94 -> Multi-Temperature SOC EKF94/3(Temperature)  "<Cell Temperature 94>"
  Bus Selector2/86 -> Multi-Temperature SOC EKF86/3(Temperature)  "<Cell Temperature 86>"
  [HVBatCell_Voltage002] -> Multi-Temperature SOC EKF2/2(Voltage)
  [HVBatCell_Voltage006] -> Multi-Temperature SOC EKF6/2(Voltage)
  Bus Selector2/77 -> Multi-Temperature SOC EKF77/3(Temperature)  "<Cell Temperature 77>"
  Multi-Temperature SOC EKF24/1(HVBat_SOCcell024) -> Bus Creator2/24  "HVBat_SOCcell024"
  Multi-Temperature SOC EKF36/1(HVBat_SOCcell036) -> Bus Creator2/36  "HVBat_SOCcell036"
  Bus Selector2/105 -> Multi-Temperature SOC EKF105/3(Temperature)  "<Cell Temperature 105>"
  Bus Selector2/102 -> Multi-Temperature SOC EKF102/3(Temperature)  "<Cell Temperature 102>"
  [HVBatCell_Voltage058] -> Multi-Temperature SOC EKF58/2(Voltage)
  Bus Selector2/11 -> Multi-Temperature SOC EKF11/3(Temperature)  "<Cell Temperature 11>"
  Bus Selector2/22 -> Multi-Temperature SOC EKF22/3(Temperature)  "<Cell Temperature 22>"
  Multi-Temperature SOC EKF21/1(HVBat_SOCcell021) -> Bus Creator2/21  "HVBat_SOCcell021"
  Bus Selector2/28 -> Multi-Temperature SOC EKF28/3(Temperature)  "<Cell Temperature 28>"
  Bus Selector2/96 -> Multi-Temperature SOC EKF96/3(Temperature)  "<Cell Temperature 66>"
  [HVBatCell_Voltage056] -> Multi-Temperature SOC EKF56/2(Voltage)
